simulink library "ee_lib_for_power_systems"  (R2025b)
settings: Solver=VariableStepAuto  StartTime=0.0  StopTime=10.0  RelTol=1e-3
undefined symbols (not defined in the file or in its folder): Alt_PolePair_Count, C3m, DiscIntNeverNeededParam, Enum1to12, L3m, Total_SM_Inertia, Ts
libraries: fl_lib x291, nesl_utility x219, ee_lib x139, custom_lib x12, $bdroot x10, ee_sl_lib x3, eeTransforms x2, eeGeneralControl x1, simulink x1
(part 11 of 11)

== system ee_lib_for_power_systems/Power Converters/Two-Level Average-Value/Subsystem4 ==
  p3 : Inport
  p2 : Inport  Port=2
  p1 : Inport  Port=3
  PS Add : fl_lib/Physical Signals/Functions/PS Add
  PS Add1 : fl_lib/Physical Signals/Functions/PS Add
  PS Gain : fl_lib/Physical Signals/Functions/PS Gain  gain=1
  PS Product2 : fl_lib/Physical Signals/Functions/PS Product
  PS Product7 : fl_lib/Physical Signals/Functions/PS Product
  PS Product8 : fl_lib/Physical Signals/Functions/PS Product
  Simulink-PS Converter : nesl_utility/Simulink-PS Converter  PhysicalDomain=network_engine_domain  SubClassName=ps_input  LeftPortType=input  RightPortType=output  PseudoPeriodic=off  Frequencies=[]  NoiseDistribution=none  NoiseParameters=[]  Unit=1  AffineConversion=off  FilteringAndDerivatives=provide  SimscapeFilterOrder=1  InputFilterTimeConstant=0.001  UdotUserProvided=0
  Simulink-PS Converter1 : nesl_utility/Simulink-PS Converter  PhysicalDomain=network_engine_domain  SubClassName=ps_input  LeftPortType=input  RightPortType=output  PseudoPeriodic=off  Frequencies=[]  NoiseDistribution=none  NoiseParameters=[]  Unit=1  AffineConversion=off  FilteringAndDerivatives=provide  SimscapeFilterOrder=1  InputFilterTimeConstant=0.001  UdotUserProvided=0
  Simulink-PS Converter2 : nesl_utility/Simulink-PS Converter  PhysicalDomain=network_engine_domain  SubClassName=ps_input  LeftPortType=input  RightPortType=output  PseudoPeriodic=off  Frequencies=[]  NoiseDistribution=none  NoiseParameters=[]  Unit=1  AffineConversion=off  FilteringAndDerivatives=provide  SimscapeFilterOrder=1  InputFilterTimeConstant=0.001  UdotUserProvided=0
  IAN : PMIOPort  Side=Left
  IBN : PMIOPort  Port=2  Side=Left
  ICN : PMIOPort  Port=3  Side=Left
  IDCN : PMIOPort  Port=4  Side=Right
  wires:
  p3/1 -> Simulink-PS Converter2/1
  p2/1 -> Simulink-PS Converter1/1
  p1/1 -> Simulink-PS Converter/1
  connections:
  PS Add1/RConn1 -- PS Gain/LConn1
  PS Gain/RConn1 -- IDCN/RConn1
  PS Product2/LConn1 -- IAN/RConn1
  PS Add1/LConn1 -- PS Product8/RConn1
  PS Add1/LConn2 -- PS Add/RConn1
  Simulink-PS Converter/RConn1 -- PS Product2/LConn2
  PS Add/LConn2 -- PS Product2/RConn1
  PS Add/LConn1 -- PS Product7/RConn1
  PS Product8/LConn1 -- ICN/RConn1
  PS Product8/LConn2 -- Simulink-PS Converter2/RConn1
  PS Product7/LConn2 -- Simulink-PS Converter1/RConn1
  PS Product7/LConn1 -- IBN/RConn1

== system ee_lib_for_power_systems/Electromechanical Machines/Vectorized Simulink Synchronous Machine (PU)/Stator side phase voltages --> qd voltages/Park Transform ==
  abc : Inport
  theta : Inport  Port=2
  Constant : Constant  Value=-2*pi/3*ones(1,no_meas)  VectorParams1D=off  SampleTime=Ts
  Constant1 : Constant  Value=2*pi/3*ones(1,no_meas)  VectorParams1D=off  SampleTime=Ts
  Constant2 : Constant  Value=0.5*ones(1,no_meas)  VectorParams1D=off  SampleTime=Ts
  Constant3 : Constant  Value=0.5*ones(1,no_meas)  VectorParams1D=off  SampleTime=Ts
  Constant4 : Constant  Value=0.5*ones(1,no_meas)  VectorParams1D=off  SampleTime=Ts
  Gain : Gain  Gain=2/3
  Gain1 : Gain  Gain=2/3
  Gain2 : Gain  Gain=2/3
  Product : Product
  Product1 : Product
  Product2 : Product
  Sin : Trigonometry
  Sin1 : Trigonometry
  Sin2 : Trigonometry  Operator=cos
  Sin3 : Trigonometry  Operator=cos
  Sin6 : Trigonometry
  Sin7 : Trigonometry  Operator=cos
  Sum{1,5..7} (4 alike) : Sum  Inputs=++|
  Sum2 : Sum  Inputs=1  CollapseMode=Specified dimension  InputSameDT=on  SaturateOnIntegerOverflow=on  OutDataTypeStr=Inherit: Same as first input
  Sum3 : Sum  Inputs=1  CollapseMode=Specified dimension  InputSameDT=on  SaturateOnIntegerOverflow=on  OutDataTypeStr=Inherit: Same as first input
  Sum4 : Sum  Inputs=1  CollapseMode=Specified dimension  InputSameDT=on  SaturateOnIntegerOverflow=on  OutDataTypeStr=Inherit: Same as first input
  Terminator : Terminator
  Vector Concatenate1 : Concatenate  NumInputs=3  Mode=Multidimensional array
  Vector Concatenate2 : Concatenate  NumInputs=3  Mode=Multidimensional array
  Vector Concatenate3 : Concatenate  NumInputs=3  Mode=Multidimensional array
  q : Outport  VectorParamsAs1DForOutWhenUnconnected=off
  d : Outport  Port=2  VectorParamsAs1DForOutWhenUnconnected=off
  wires:
  Gain/1 -> d/1
  Gain1/1 -> q/1
  Gain2/1 -> Terminator/1
  Sum2/1 -> Gain/1
  Sum4/1 -> Gain2/1
  Sum3/1 -> Gain1/1
  Product2/1 -> Sum4/1
  Product1/1 -> Sum3/1
  Product/1 -> Sum2/1
  Vector Concatenate1/1 -> Product/2
  Vector Concatenate2/1 -> Product1/2
  Vector Concatenate3/1 -> Product2/2
  Constant3/1 -> Vector Concatenate3/3
  Sin7/1 -> Vector Concatenate2/3
  Sin6/1 -> Vector Concatenate1/3
  Constant2/1 -> Vector Concatenate3/2
  Constant4/1 -> Vector Concatenate3/1
  Sin2/1 -> Vector Concatenate2/1
  Sin3/1 -> Vector Concatenate2/2
  Sin1/1 -> Vector Concatenate1/2
  Sin/1 -> Vector Concatenate1/1
  abc/1 -> Product/1, Product1/1, Product2/1
  Sum5/1 -> Sin1/1
  Sum6/1 -> Sin6/1
  Constant/1 -> Sum1/2, Sum5/2
  Constant1/1 -> Sum7/2, Sum6/2
  Sum1/1 -> Sin3/1
  Sum7/1 -> Sin7/1
  theta/1 -> Sum6/1, Sum5/1, Sin2/1, Sum1/1, Sin/1, Sum7/1
